simulink model "HDVSim_BEV_WPT_Truck"  (R2018b)
settings: Solver=ode3  StartTime=0.0  StopTime=inf  FixedStep=sample_time  MaxStep=0.1  RelTol=1e-3  EnableMultiTasking=on
model workspace (MAT data in the model file):
  Anschaffungskosten = [3707764736; 2; 1; 1; 1; 1]
  Bat.Useable_range = 1
  Bat.Useable_capacity = 400
  Bat.Type = 2
  Bat.SOC_start = 1
  Bat.Voltage = 800
  Bat.Efficiency_power_electronics = 0.95
  Bat.Charge_cycles = 2274.15994574
  DrvTrn = 12
  Eigenschaften = [3707764736; 2; 1; 1; 2; 2]
  Electric_Truck = 1
  Elektro_LKW = 1
  Fahrzyklus = 2
  FuelCell.P_nom = 0
  Fueltype = 12
  Gewichte = [3707764736; 2; 1; 1; 3; 3]
  Hybrid_LKW = 1
  Hybrid_Truck = 1
  IZ12 = 3600
  Kraftstoff = 12
  SOC_T_EM_completely_available = 0.5
  SOC_addition = 0.4
  SOC_target = 0.85
  Sailing_without_drag_torque = 1
  TCO = TCO
  TCO.Verhaeltnis_Anh_Lkw = [1 1]
  TCO.Kaufpreis = [141995 59191]
  TCO.Preisanstieg_Wiederbeschaffung = [3.5 3.5]
  TCO.Wiederbeschaffungsneupreis = [96696 54183]
  TCO.Restwert = [42599 0]
  TCO.CNG_Verbrauch = 0
  TCO.LNG_Verbrauch = 0
  TCO.Wasserstoffverbrauch = 0
  TCO.Reifenkosten_Satz = [2700 5008]
  TCO.Abschreibungswert = [118864 81874]
  TCO.Mautsatz = 0
  TCO.Tagesarbeitszeit = 8
  TCO.Sonstige_Arbeiten_Tag = 0.5
  TCO.Bruttostundenlohn_Fahrer = 13
  TCO.Einsatztage_Jahr = 240
  TCO.Jahresbruttolohn_Fahrer = 24960
  TCO.Personalfaktor = 1.3
  TCO.Jahresbruttolohn_FZG = 32448
  TCO.Sozialaufwendungen_Proz = 25.75
  TCO.Sozialaufwendungen_EUR = 8355
  TCO.Aushilfsfahrer = 0
  TCO.Spesen_Tag = 12
  TCO.Spesen_Jahr = 2880
  TCO.Fahrerkosten_Sonstige = 0
  TCO.Fahrpersonalkosten_Summe = 43683
  TCO.Dieselpreis = 0.95
  TCO.Dieselpreis_Fremd = 1
  TCO.Adbluepreis = 0.68
  TCO.CNGpreis = 0.88
  TCO.LNGpreis = 0.81
  TCO.Strompreis = 0.12
  TCO.Wasserstoffpreis = 9
  TCO.Verzinsung_Umlaufvermoegen = 8.4
  TCO.Verzinsung_Anlagevermoegen = 8.4
  TCO.Verwaltungskosten = 10.7
  TCO.Fahrtstrecke = 358.120895046
  TCO.Anteil_Mautstrecke = 75
  TCO.Beladezeit_Gespann = 0.25
  TCO.Entladezeit_Gespann = 0.25
  TCO.Anteil_Leerfahrten = 0
  TCO.Durchschnittsgeschwindigkeit = 75.7215565703
  TCO.Tageslenkzeit = 6.78
  TCO.Jahresfahrleistung = [123214 123214]
  TCO.Nutzungsdauer = [6 12]
  TCO.Reifenfahrleistung = [140000 170000]
  TCO.Anteil_Eigentankung_Diesel = 75
  TCO.Schmierstoffverbauch = 1
  TCO.Adblueverbrauch = 4
  TCO.Afa_zeitabhaengig = [100 100]
  TCO.Afa_leistungsabhaengig = [0 0]
  TCO.Umlaufvermoegen = [6000 1400]
  TCO.Steuern = [556 373]
  TCO.Versicherung_Kfz = [6298 677]
  TCO.Versicherung_Transport = 1000
  TCO.Kommunikation = 600
  TCO.Reparaturen_Wartung_Pflege = [0.13 0.025]
  TCO.Abschreibung_zeitabh = [19811 6823]
  TCO.Umlaufvermoegen_verzinst = 622
  TCO.Anlagevermoegen_verzinst = [5964 2486]
  TCO.Versicherung = [7298 677]
  TCO.Summe_Fzg_Kosten = [34851 10359]
  TCO.Summe_fixe_Kosten = [78534 10359]
  TCO.Abschreibung_leistungsabh = [0 0]
  TCO.Reparaturkosten = [16018 3080]
  TCO.Reifenkosten = [2376 3630]
  TCO.Lebensdauer_Batteriepack = 4.74658995879
  TCO.Anschaffungspreis_Akkupack = 65171.4590287
  TCO.Verschleisskosten_Batteriepack = 65171
  TCO.Summe_variable_Kosten = [83565 6710]
  TCO.Summe_fixe_variable_Kosten = [162099 17069]
  TCO.Gemeinkosten = 21468
  TCO.Mautkosten = 0
  TCO.Gesamtkosten = 200636
  TCO.Ergebnis = table
  TCO.Ergebnis.data = {{[]; 26634; 622; 8450; 929; 7975; 600; 45210; 43683; 88893; []; 0; 0; 0; 19098; 0; 6006; 90275; []; 179168; 21468; 0; 200636}, {[]; 0.216160501242; 0.00504812764783; 0.0685798691707; 0.00753972762835; 0.0647247877676; 0.00486957650916; 0.366922589965; 0.35452951775; 0.721452107715; []; 0; 0; 0; 0.154998620287; 0; 0.0487444608567; 0.732668365608; []; 1.45412047332; 0.174233447498; 0; 1.62835392082}, {[]; []; []; []; []; []; []; []; []; []; []; []; []; []; []; []; []; []; []; []; []; []; []}, {'#cccccc'; '#9DFF00'; '#CEFF00'; '#FFFF00'; '#FFDF00'; '#FFB900'; '#FF9200'; '#FF5D00'; '#FF7F7F'; '#FF2900'; '#cccccc'; '#00FF85'; '#00FFC6'; '#00FFF2'; '#00FFFF'; '#00DBFF'; '#00B3FF'; '#0088FF'; '#cccccc'; '#B100FF'; '#FF00FF'; '#FF00C0'; '#FF0070'}, [0; 1; 1; 1; 1; 1; 1; 0; 1; 0; 0; 1; 1; 1; 1; 1; 1; 0; 0; 0; 1; 1; 0]}
  TCO.Ergebnis.ndims = 2
  TCO.Ergebnis.nrows = 23
  TCO.Ergebnis.rownames = {'Zeitabh_Fzg_Kosten'; 'Abschreibung_zeitabh'; 'Umlaufvermoegen_verzinst'; 'Anlagevermoegen_verzinst'; 'Steuern'; 'Versicherung'; 'Kommunikation'; 'Summe_Fzg_Kosten'; 'Fahrpersonalkosten_Summe'; 'Summe_fixe_Kosten'; 'Leistungsabh_Fzg_Kosten'; 'Abschreibung_leistungsabh'; 'Kraftstoffkosten'; 'Adbluekosten'; 'Reparaturkosten'; 'Schmierstoffkosten'; 'Reifenkosten'; 'Summe_variable_Kosten'; 'Summe_Kosten'; 'Summe_fixe_variable_Kosten'; 'Gemeinkosten'; 'Mautkosten'; 'Gesamtkosten'}
  TCO.Ergebnis.nvars = 5
  TCO.Ergebnis.varnames = {'Euro_Jahr', 'Euro_km', 'Prozent', 'Cellcolor', 'Diagramm'}
  TCO.Ergebnis.props.CustomProps = struct()
  TCO.Ergebnis.props.VariableCustomProps = struct()
  TCO.Ergebnis.props.versionSavedFrom = 3
  TCO.Ergebnis.props.minCompatibleVersion = 1
  TCO.Ergebnis.props.incompatibilityMsg = ''
  TCO.Ergebnis.props.VersionSavedFrom = 3
  TCO.Ergebnis.props.Description = ''
  TCO.Ergebnis.props.DimensionNames = {'Row', 'Variables'}
  TCO.Ergebnis.props.UserData = []
  TCO.Ergebnis.props.VariableDescriptions = {}
  TCO.Ergebnis.props.VariableUnits = {}
  TCO.Ergebnis.props.VariableContinuity = {}
  TCO_Anhaenger = 1
  TCO_Trailer = 1
  T_distance_LPS_down = 250
  T_distance_LPS_up = 250
  Temp1 = [1000 1100 1200 1300 1400 1500 1600 1700 1800 1900 2000 2100 2200 2300 2400 2500 2600 2700 2800 2900 3000 3100 3200 3300 3400 3500 3600 3700 3800 3900 4000 4100 4200 4300 4400 4500 4600 4700 4800 4900 5000]
  VSim.Display = 3
  VSim.Entwurf = 0
  VSim.Opt = 0
  VSim.v_t = [0 0 0 0 0 0 7.99755670495 16.5932528737 22.7161932573 27.4198466579 27.9147182661 29.6860764389 33.4325730161 36.7505814021 39.7508189942 42.5028973365 44.7631603047 44.2850927904 46.12750995 48.4837783408 50.7030690616 52.8034153875 54.7991287178 56.7017938485 58.520948941 60.2645598986 61.9393623324 63.551113455 65.1047825506 66.6046953402 68.0546450626 69.4579815644 70.8176809608 72.1364027813 72.1303435542 72.2830929427 73.5794997033 74.8382617013 76.0614012431 77.2507716609 78.4080631952 79.5348241022 80.6324765407 81.7023309876 82.7455984026 83.7634008372 84.7567809142 85.7267097376 86.6740926498 86.812753067 86.4835070605 86.3865018146 86.3615004533]
  Vehicle = 'BEV_WPT'
  Vorausschauender_Tempomat = 1
  WPT.Voltage = 800
  WPT.SOC_target = 0.8
  WPT.SOC_electric_only = 0.3
  WPT.P_max = 200
  WPT.eta = 0.88
  WPT.expansion = 0.43
  acquisition_costs = [3707764736; 2; 1; 1; 3; 3]
  addition_for_critical_slope = 0.01
  ambient.gravity = 9.80665
  ambient.air_density = 1.1883
  ambient.friction_coeff = 0.8
  auxiliary_consumers.onBattery = 0
  auxiliary_consumers.power = 3500
  critical_altitude_difference = 50
  cycle.distance = [0 1 2 3 4 5 6 7 8 9 10 11 ... (358139 elements, 358139x1)]
  cycle.speed = [21.8055555556 21.8055555556 21.8055555556 21.8055555556 21.8055555556 21.8055555556 21.8055555556 21.8055555556 21.8055555556 21.8055555556 21.8055555556 21.8055555556 ... (358139 elements, 358139x1)]
  cycle.slope = [-0.0160892295393 -0.0161214571277 -0.0161536847161 -0.0161859123044 -0.0162181398928 -0.0162503674812 -0.0162825950696 -0.016314822658 -0.0163470502464 -0.0163792778348 -0.0164115054232 -0.0164437330115 ... (358139 elements, 358139x1)]
  cycle.stop_time = [0 0 0 0 0 0 0 0 0 0 0 0 ... (358139 elements, 358139x1)]
  cycle.altitude = [558.98391077 558.967789313 558.951635629 558.935449716 558.919231576 558.902981209 558.886698614 558.870383791 558.854036741 558.837657463 558.821245958 558.804802225 ... (358139 elements, 358139x1)]
  cycle.stop_end = 0
  cycle.stop_start = 0
  cycle.speed_init = 0
  cycle.delta12 = [0 0 0 0 0 0 0 0 0 0 0 0 ... (358139 elements, 1x358139)]
  cycle.delta23 = [0 0 0 0 0 0 0 0 0 0 0 0 ... (358139 elements, 1x358139)]
  dcycle = 2
  distance_1_PPC = 175
  distance_2_PPC = 200
  distance_PPC_altitude_difference = 10000
  driver.controller_proportional_gain = 230000
  driver.controller_integral_gain = 3000
  driver.slew_rate_throttle_in = 2
  driver.slew_rate_throttle_out = -2
  driver.slew_rate_brake_in = 2
  driver.slew_rate_brake_out = -2
  em.M_max = 1563
  em.n_max = 10000
  em.n_eck = 2200
  em.P_max = 360.089349954
  em.shift_parameter.n_pref = 2068
  em.shift_parameter.n2 = 462
  em.shift_parameter.n6 = 8000
  em.shift_parameter.M_max = 1563
  em.fuel.co2_per_kwh = 504
  em.speed = [0 10 20 30 40 50 60 70 80 90 100 110 ... (1001 elements, 1x1001)]
  em.trq = [1563 1563 1563 1563 1563 1563 1563 1563 1563 1563 1563 1563 ... (1001 elements, 1x1001)]
  em.Type = 2
  em.efficiency.characteristic_map = [0.001 0.001 0.001 0.001 0.001 0.001 0.001 0.001 0.001 0.001 0.001 0.001 ... (20301 elements, 101x201)]
  em.efficiency.speed = [0 50 100 150 200 250 300 350 400 450 500 550 600 650 700 750 800 850 900 950 1000 1050 1100 1150 1200 1250 1300 1350 1400 1450 1500 1550 1600 1650 1700 1750 1800 1850 1900 1950 2000 2050 2100 2150 2200 2250 2300 2350 2400 2450 2500 2550 2600 2650 2700 2750 2800 2850 2900 2950 3000 3050 3100 3150 3200 3250 3300 3350 3400 3450 3500 3550 3600 3650 3700 3750 3800 3850 3900 3950 4000 4050 4100 4150 4200 4250 4300 4350 4400 4450 4500 4550 4600 4650 4700 4750 4800 4850 4900 4950 5000 5050 5100 5150 5200 5250 5300 5350 5400 5450 5500 5550 5600 5650 5700 5750 5800 5850 5900 5950 6000 6050 6100 6150 6200 6250 6300 6350 6400 6450 6500 6550 6600 6650 6700 6750 6800 6850 6900 6950 7000 7050 7100 7150 7200 7250 7300 7350 7400 7450 7500 7550 7600 7650 7700 7750 7800 7850 7900 7950 8000 8050 8100 8150 8200 8250 8300 8350 8400 8450 8500 8550 8600 8650 8700 8750 8800 8850 8900 8950 9000 9050 9100 9150 9200 9250 9300 9350 9400 9450 9500 9550 9600 9650 9700 9750 9800 9850 9900 9950 10000]
  em.efficiency.torque = [0 15.63 31.26 46.89 62.52 78.15 93.78 109.41 125.04 140.67 156.3 171.93 187.56 203.19 218.82 234.45 250.08 265.71 281.34 296.97 312.6 328.23 343.86 359.49 375.12 390.75 406.38 422.01 437.64 453.27 468.9 484.53 500.16 515.79 531.42 547.05 562.68 578.31 593.94 609.57 625.2 640.83 656.46 672.09 687.72 703.35 718.98 734.61 750.24 765.87 781.5 797.13 812.76 828.39 844.02 859.65 875.28 890.91 906.54 922.17 937.8 953.43 969.06 984.69 1000.32 1015.95 1031.58 1047.21 1062.84 1078.47 1094.1 1109.73 1125.36 1140.99 1156.62 1172.25 1187.88 1203.51 1219.14 1234.77 1250.4 1266.03 1281.66 1297.29 1312.92 1328.55 1344.18 1359.81 1375.44 1391.07 1406.7 1422.33 1437.96 1453.59 1469.22 1484.85 1500.48 1516.11 1531.74 1547.37 1563]
  em_M_max = 1563
  engine.shift_parameter.n_lo = 978
  engine.shift_parameter.n_pref = 1339
  engine.M_max = 0
  engine.number = 4
  final_drive.ratio = 10.0500050682
  final_drive.trq_eff = 0.98
  ifElectric = 1
  ifOptimized = 1
  look_ahead_PPC = 250
  max_distance = 358138
  name = 'Verbrauchssimulation_Elektro_WPT_LKW'
  propertie = [3707764736; 2; 1; 1; 4; 4]
  prospective_cruise_control = 1
  sample_time = 0.1
  tank.v_diesel = 0
  tank.v_lng = 0
  tank.v_cng = 0
  tank.m_h2 = 0
  tires.radius = 0.4465
  tires.roll_drag_coeff.total = 0.0043
  transmission.init_gear = 1
  transmission.shift_time = 1
  transmission.ratios = [2.66167387947 1.631463723 1 0.612946512939]
  transmission.trq_eff = [0.96 0.97 0.98 0.99]
  transmission.lamda = [1.0669857778 1.02376767201 1.01308044742 1.00911164176]
  transmission.z = 4
  v_PPC_delta = 2.77777777778
  v_max_electrical_drive_only = 13.8888888889
  vehicle.long_truck = 0
  vehicle.payload = 19300
  vehicle.frontal_area = 10.2
  vehicle.air_drag_coeff = 0.53
  vehicle.Fx_max = 90221.18
  vehicle.F_brake_max = 309890.14
  vehicle.mass = 13863.4845097
  vehicle_F_brake_max = 309890.14
  weights = weights
  weights.m_Gearbox = 146.265985714
  weights.m_Retarder = 82
  weights.m_Battery = 1987.57763975
  weights.m_EM = 400.099277727
  weights.m_PwrElectr = 33.3416064773
  weights.m_Charger = 591
  weights.m_Trailer = 5400
  weights.m_Total = 8463.48450967
  weights.m_Base = 5223.2
  weights.Electric = 1
  weights.Hybrid = 1
  weights.max_Payload = 27136.5154903
  weights.m_Max = 41000
  x = [1563 13 1 400 10.0500050682 4.34242437682 3 1 0 978 1339 2 2 0.8 0.3]
values from neighbouring files (symbols this model uses):
  transmission = Transmission_gearing(15.86, 7, 0, 0, final_drive.ratio)    [Parameterizing.m]
undefined symbols (not defined in the file or in its folder): Ion, Lithium
libraries: simulink x22, powerlib x4, electricdrivelib x1
(part 1 of 3)
settings Model: Creator=Alexander Suessmann  LastModifiedBy=ga73rep
settings DataIOCC: SaveFormat=Array  SignalLoggingSaveFormat=ModelDataLogs  SaveOutput=off  SignalLogging=off  DSMLogging=off  SignalLoggingName=datalog
settings OptimizationCC: ExpressionDepthLimit=2147483647  InitFltsAndDblsToZero=on  LifeSpan=inf
settings DebuggingCC: UnderspecifiedInitializationDetection=Classic  MergeDetectMultiDrivingBlocksExec=none  SolverPrmCheckMsg=warning  MultiTaskDSMMsg=warning  MultiTaskCondExecSysMsg=none  FcnCallInpInsideContextMsg=warning  AllowSymbolicDim=off  StateNameClashWarn=warning  LibraryContextMissingDiagnostic=none  SFUnexpectedBacktrackingDiag=warning  SFNoUnconditionalDefaultTransitionDiag=warning  SFExecutionAtInitializationDiag=none
settings HardwareCC: ProdBitPerPointer=32  ProdBitPerSizeT=32  ProdBitPerPtrDiffT=32  ProdLargestAtomicFloat=None  ProdIntDivRoundTo=Undefined  ProdEndianess=Unspecified  ProdWordSize=32  ProdHWDeviceType=32-bit Generic
settings ModelReferenceCC: PropagateSignalLabelsOutOfModel=off
settings SFSimCC: SimUseLocalCustomCode=off
settings RTWCC: RTWUseLocalCustomCode=off
settings CodeAppCC: StateflowObjectComments=on
settings GRTTargetCC: TargetLangStandard=C89/C90 (ANSI)  GeneratePreprocessorConditionals=Disable all  CombineOutputUpdateFcns=off  GRTInterface=on
settings ConfigComp: CovMetricSettings=dw  CovHtmlReporting=on  CovSaveCumulativeToWorkspaceVar=on  CovSaveSingleToWorkspaceVar=on  CovExternalEMLEnable=off  CovSFcnEnable=off
settings SimscapeCC: SimscapeNormalizeSystem=off

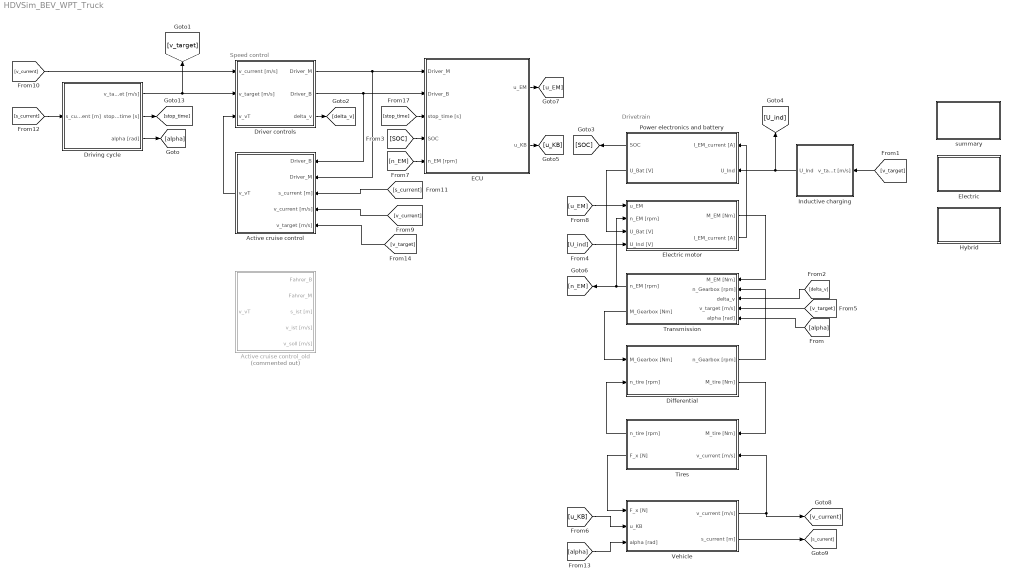
== system HDVSim_BEV_WPT_Truck ==
  note: Drivetrain
  note: Speed control
  Active cruise control : Subsystem
  Active cruise control_old : Subsystem  Commented=on
  Differential : Subsystem
  Driver controls : Subsystem
  Driving cycle : Subsystem
  ECU : Subsystem
  Electric : Subsystem
  Electric motor : Subsystem
  Hybrid : Subsystem
  Inductive charging : Subsystem
  Power electronics and battery : Subsystem
  Tires : Subsystem
  Transmission : Subsystem
  Vehicle : Subsystem
  summary : Subsystem
  wires:
  Vehicle/1(v_current [m/s]) -> [v_current], Tires/2(v_current [m/s])
  Active cruise control/1(v_vT) -> Driver controls/3(v_vT)
  Driver controls/1(Driver_M) -> Active cruise control/2(Driver_M), ECU/1(Driver_M)
  Driver controls/2(Driver_B) -> Active cruise control/1(Driver_B), ECU/2(Driver_B)
  [v_target] -> Inductive charging/1(v_target [m/s])
  Inductive charging/1(U_Ind) -> Power electronics and battery/2(U_Ind), [U_ind]
  Transmission/2(M_Gearbox [Nm]) -> Differential/1(M_Gearbox [Nm])
  Differential/1(n_Gearbox [rpm]) -> Transmission/2(n_Gearbox [rpm])
  Electric motor/1(M_EM [Nm]) -> Transmission/1(M_EM [Nm])
  Electric motor/2(I_EM_current [A]) -> Power electronics and battery/1(I_EM_current [A])
  [alpha] -> Transmission/5(alpha [rad])
  [delta_v] -> Transmission/3(delta_v)
  Power electronics and battery/1(SOC) -> [SOC]
  [SOC] -> ECU/4(SOC)
  Power electronics and battery/2(U_Bat [V]) -> Electric motor/3(U_Bat [V])
  Transmission/1(n_EM [rpm]) -> [n_EM], Electric motor/2(n_EM [rpm])
  [U_ind] -> Electric motor/4(U_Ind [V])
  [v_target] -> Transmission/4(v_target [m/s])
  Differential/2(M_tire [Nm]) -> Tires/1(M_tire [Nm])
  Tires/1(n_tire [rpm]) -> Differential/2(n_tire [rpm])
  Tires/2(F_x [N]) -> Vehicle/1(F_x [N])
  ECU/2(u_KB) -> [u_KB]
  [u_KB] -> Vehicle/2(u_KB)
  [n_EM] -> ECU/5(n_EM [rpm])
  ECU/1(u_EM) -> [u_EM]
  [u_EM] -> Electric motor/1(u_EM)
  [v_current] -> Active cruise control/4(v_current [m/s])
  [v_current] -> Driver controls/1(v_current [m/s])
  Vehicle/2(s_current [m]) -> [s_current]
  [s_current] -> Active cruise control/3(s_current [m])
  [s_current] -> Driving cycle/1(s_current [m])
  Driving cycle/3(alpha [rad]) -> [alpha]
  [alpha] -> Vehicle/3(alpha [rad])
  Driving cycle/1(v_target [m/s]) -> [v_target], Driver controls/2(v_target [m/s])
  [v_target] -> Active cruise control/5(v_target [m/s])
  Driving cycle/2(stop_time [s]) -> [stop_time]
  [stop_time] -> ECU/3(stop_time [s])
  Driver controls/3(delta_v) -> [delta_v]

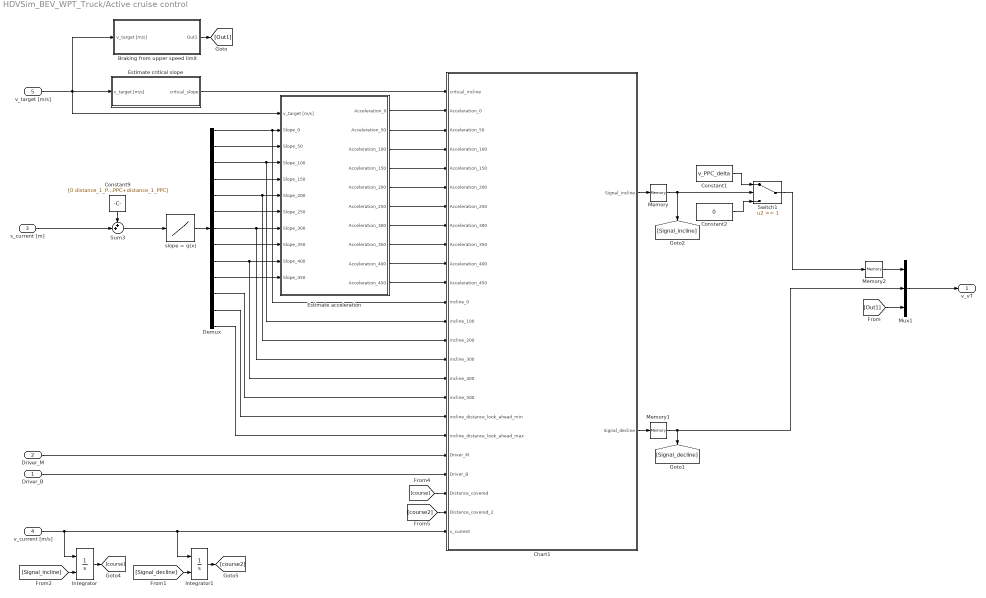
== system HDVSim_BEV_WPT_Truck/Active cruise control ==
  Driver_B : Inport
  Driver_M : Inport  Port=2
  s_current [m] : Inport  Port=3
  v_current [m/s] : Inport  Port=4
  v_target [m/s] : Inport  Port=5
  Braking from upper speed limit : Subsystem
  Chart1 : Stateflow chart  TreatAsAtomicUnit=on
  Constant1 : Constant  Value=v_PPC_delta
  Constant2 : Constant  Value=0
  Constant9 : Constant  Value=[0 distance_1_PPC/5 2*distance_1_PPC/5 3*distance_1_PPC/5 4*distance_1_PPC/5 distance_1_PPC 6*distance_1_PPC/5 7*distance_1_PPC/5 8*distance_1_PPC/5 9*distance_1_PPC/5 10*distance_1_PPC/5 look_ahead_PPC look_ahead_PPC+distance_1_PPC]
  Demux : Demux  Outputs=13
  Estimate acceleration : Subsystem
  Estimate critical slope : Subsystem
  Integrator : Integrator  ExternalReset=rising
  Integrator1 : Integrator  ExternalReset=rising
  Memory : Memory
  Memory1 : Memory
  Memory2 : Memory
  Mux1 : Mux  Inputs=3
  Sum3 : Sum  Inputs=++|
  Switch1 : Switch  Threshold=1
  slope = q(x) : Lookup  InputValues=cycle.distance  Table=cycle.slope  LookUpMeth=Interpolation-Use End Values
  v_vT : Outport
  wires:
  slope = q(x)/1 -> Demux/1
  Demux/1 -> Chart1/12(incline_0), Estimate acceleration/2(Slope_0)
  Estimate critical slope/1(critical_slope) -> Chart1/1(critical_incline)
  Chart1/1(Signal_incline) -> Memory/1
  v_current [m/s]/1 -> Integrator/1, Chart1/24(v_current), Integrator1/1
  Memory/1 -> [Signal_incline], Switch1/2
  Mux1/1 -> v_vT/1
  Constant9/1 -> Sum3/1
  Sum3/1 -> slope = q(x)/1
  Estimate acceleration/1(Acceleration_0) -> Chart1/2(Acceleration_0)
  Estimate acceleration/2(Acceleration_50) -> Chart1/3(Acceleration_50)
  Estimate acceleration/3(Acceleration_100) -> Chart1/4(Acceleration_100)
  Estimate acceleration/4(Acceleration_150) -> Chart1/5(Acceleration_150)
  Estimate acceleration/5(Acceleration_200) -> Chart1/6(Acceleration_200)
  Estimate acceleration/6(Acceleration_250) -> Chart1/7(Acceleration_250)
  Estimate acceleration/7(Acceleration_300) -> Chart1/8(Acceleration_300)
  Estimate acceleration/8(Acceleration_350) -> Chart1/9(Acceleration_350)
  Chart1/2(Signal_decline) -> Memory1/1
  Memory1/1 -> Mux1/2, [Signal_decline]
  Driver_B/1 -> Chart1/21(Driver_B)
  Estimate acceleration/9(Acceleration_400) -> Chart1/10(Acceleration_400)
  Estimate acceleration/10(Acceleration_450) -> Chart1/11(Acceleration_450)
  Driver_M/1 -> Chart1/20(Driver_M)
  Demux/2 -> Estimate acceleration/3(Slope_50)
  Demux/3 -> Chart1/13(incline_100), Estimate acceleration/4(Slope_100)
  Demux/4 -> Estimate acceleration/5(Slope_150)
  Demux/5 -> Chart1/14(incline_200), Estimate acceleration/6(Slope_200)
  Demux/6 -> Estimate acceleration/7("Slope_250 ")
  Demux/7 -> Chart1/15(incline_300), Estimate acceleration/8(Slope_300)
  Demux/8 -> Estimate acceleration/9(Slope_350)
  Demux/9 -> Chart1/16(incline_400), Estimate acceleration/10(Slope_400)
  Demux/10 -> Estimate acceleration/11(Slope_450)
  Demux/12 -> Chart1/18(incline_distance_look_ahead_min)
  Demux/13 -> Chart1/19(incline_distance_look_ahead_max)
  v_target [m/s]/1 -> Braking from upper speed limit/1(v_target [m/s]), Estimate acceleration/1(v_target [m/s]), Estimate critical slope/1(v_target [m/s])
  Memory2/1 -> Mux1/1
  Constant1/1 -> Switch1/1
  Constant2/1 -> Switch1/3
  Demux/11 -> Chart1/17(incline_500)
  Switch1/1 -> Memory2/1
  s_current [m]/1 -> Sum3/2
  [Signal_decline] -> Integrator1/2
  Integrator1/1 -> [course2]
  Integrator/1 -> [course]
  [Signal_incline] -> Integrator/2
  [course] -> Chart1/22(Distance_covered)
  [course2] -> Chart1/23(Distance_covered_2)
  Braking from upper speed limit/1(Out1) -> [Out1]
  [Out1] -> Mux1/3

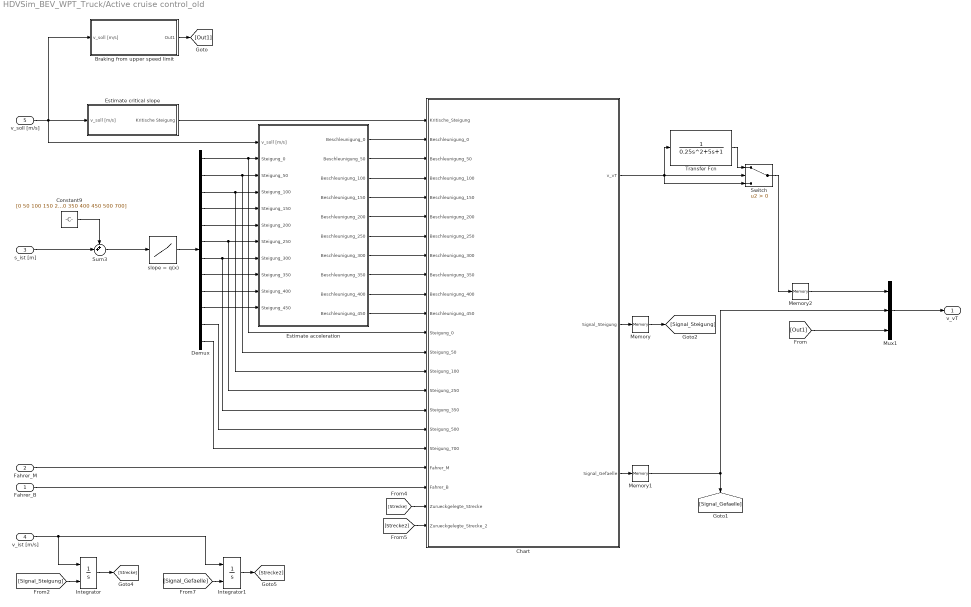
== system HDVSim_BEV_WPT_Truck/Active cruise control_old ==
  Fahrer_B : Inport
  Fahrer_M : Inport  Port=2
  s_ist [m] : Inport  Port=3
  v_ist [m/s] : Inport  Port=4
  v_soll [m/s] : Inport  Port=5
  Braking from upper speed limit : Subsystem
  Chart : Stateflow chart  TreatAsAtomicUnit=on
  Constant9 : Constant  Value=[0 50 100 150 200 250 300 350 400 450 500 700]
  Demux : Demux  Outputs=12
  Estimate acceleration : Subsystem
  Estimate critical slope : Subsystem
  Integrator : Integrator  ExternalReset=rising
  Integrator1 : Integrator  ExternalReset=rising
  Memory : Memory
  Memory1 : Memory
  Memory2 : Memory
  Mux1 : Mux  Inputs=3
  Sum3 : Sum  Inputs=++|
  Switch : Switch  Criteria=u2 > Threshold
  Transfer Fcn : TransferFcn  Denominator=[0.25 5 1]
  slope = q(x) : Lookup  InputValues=cycle.distance  Table=cycle.slope  LookUpMeth=Interpolation-Use End Values
  v_vT : Outport
  wires:
  slope = q(x)/1 -> Demux/1
  Demux/1 -> Estimate acceleration/2(Steigung_0), Chart/12(Steigung_0)
  Estimate critical slope/1(Kritische Steigung) -> Chart/1(Kritische_Steigung)
  Chart/2(Signal_Steigung) -> Memory/1
  Mux1/1 -> v_vT/1
  Constant9/1 -> Sum3/1
  s_ist [m]/1 -> Sum3/2
  Sum3/1 -> slope = q(x)/1
  Estimate acceleration/1(Beschleunigung_0) -> Chart/2(Beschleunigung_0)
  Estimate acceleration/2(Beschleunigung_50) -> Chart/3(Beschleunigung_50)
  Estimate acceleration/3(Beschleunigung_100) -> Chart/4(Beschleunigung_100)
  Estimate acceleration/4(Beschleunigung_150) -> Chart/5(Beschleunigung_150)
  Estimate acceleration/5(Beschleunigung_200) -> Chart/6(Beschleunigung_200)
  Estimate acceleration/6(Beschleunigung_250) -> Chart/7(Beschleunigung_250)
  Estimate acceleration/7(Beschleunigung_300) -> Chart/8(Beschleunigung_300)
  Estimate acceleration/8(Beschleunigung_350) -> Chart/9(Beschleunigung_350)
  Chart/3(Signal_Gefaelle) -> Memory1/1
  Memory1/1 -> [Signal_Gefaelle], Mux1/2
  Fahrer_B/1 -> Chart/20(Fahrer_B)
  Estimate acceleration/9(Beschleunigung_400) -> Chart/10(Beschleunigung_400)
  Estimate acceleration/10(Beschleunigung_450) -> Chart/11(Beschleunigung_450)
  Fahrer_M/1 -> Chart/19(Fahrer_M)
  Demux/2 -> Estimate acceleration/3(Steigung_50), Chart/13(Steigung_50)
  Demux/3 -> Estimate acceleration/4(Steigung_100), Chart/14(Steigung_100)
  Demux/4 -> Estimate acceleration/5(Steigung_150)
  Demux/5 -> Estimate acceleration/6(Steigung_200)
  Demux/6 -> Estimate acceleration/7("Steigung_250 "), Chart/15(Steigung_250)
  Demux/7 -> Estimate acceleration/8(Steigung_300), Chart/16(Steigung_350)
  Demux/8 -> Estimate acceleration/9(Steigung_350)
  Demux/9 -> Estimate acceleration/10(Steigung_400)
  Demux/10 -> Estimate acceleration/11(Steigung_450)
  Demux/11 -> Chart/17(Steigung_500)
  Demux/12 -> Chart/18(Steigung_700)
  v_soll [m/s]/1 -> Braking from upper speed limit/1(v_soll [m/s]), Estimate acceleration/1(v_soll [m/s]), Estimate critical slope/1(v_soll [m/s])
  Memory2/1 -> Mux1/1
  Switch/1 -> Memory2/1
  Chart/1(v_vT) -> Switch/3, Switch/2, Transfer Fcn/1
  Transfer Fcn/1 -> Switch/1
  Braking from upper speed limit/1(Out1) -> [Out1]
  [Out1] -> Mux1/3
  Memory/1 -> [Signal_Steigung]
  v_ist [m/s]/1 -> Integrator1/1, Integrator/1
  Integrator/1 -> [Strecke]
  [Signal_Gefaelle] -> Integrator1/2
  [Signal_Steigung] -> Integrator/2
  Integrator1/1 -> [Strecke2]
  [Strecke] -> Chart/21(Zurueckgelegte_Strecke)
  [Strecke2] -> Chart/22(Zurueckgelegte_Strecke_2)

== system HDVSim_BEV_WPT_Truck/Differential ==
  note: Effective wheel torque
  M_Gearbox [Nm] : Inport
  n_tire [rpm] : Inport  Port=2
  Differential efficiency : Constant  Value=final_drive.trq_eff
  Differential gear ratio : Constant  Value=final_drive.ratio
  M' = M*i*eta : Product  Inputs=***
  Product : Product  Inputs=/  RndMeth=Zero
  Switch : Switch  ZeroCross=off
  n' *i = n : Product  RndMeth=Zero
  n_Gearbox [rpm] : Outport
  M_tire [Nm] : Outport  Port=2
  wires:
  M_Gearbox [Nm]/1 -> M' = M*i*eta/1, Switch/2
  n_tire [rpm]/1 -> n' *i = n/2
  Differential gear ratio/1 -> n' *i = n/1, M' = M*i*eta/3
  n' *i = n/1 -> n_Gearbox [rpm]/1
  M' = M*i*eta/1 -> M_tire [Nm]/1
  Differential efficiency/1 -> Product/1, Switch/1
  Product/1 -> Switch/3
  Switch/1 -> M' = M*i*eta/2

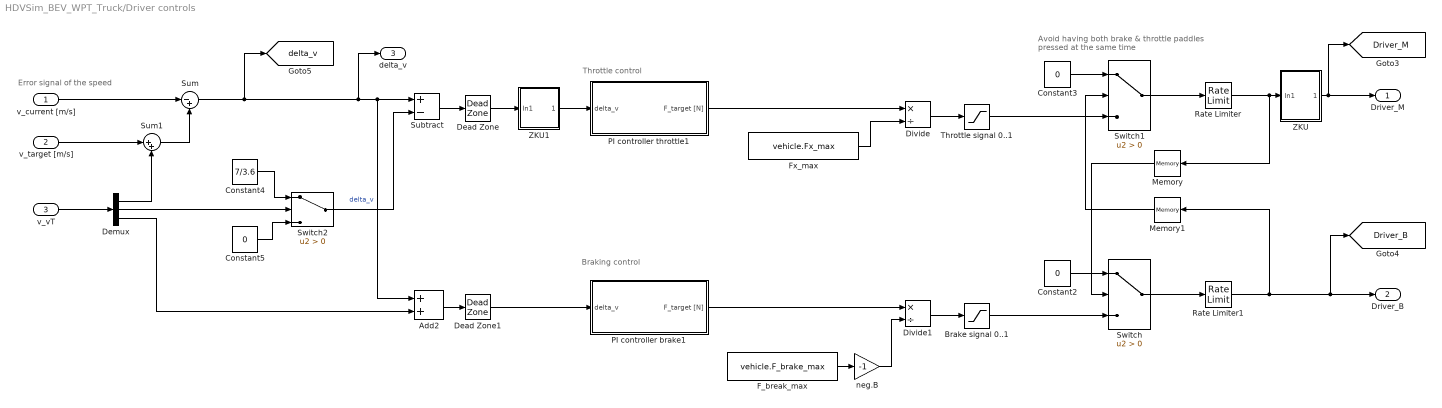
== system HDVSim_BEV_WPT_Truck/Driver controls ==
  note: Error signal of the speed
  note: Throttle control
  note: Braking control
  note: Avoid having both brake & throttle
      | paddles pressed at the same time
  v_current [m/s] : Inport
  v_target [m/s] : Inport  Port=2
  v_vT : Inport  Port=3
  Add2 : Sum
  Brake signal 0..1 : Saturate  UpperLimit=1  LowerLimit=0  ZeroCross=off
  Constant2 : Constant  Value=0
  Constant3 : Constant  Value=0
  Constant4 : Constant  Value=7/3.6
  Constant5 : Constant  Value=0
  Dead Zone : DeadZone  LowerValue=-0.1/3.6  UpperValue=0.1/3.6  ZeroCross=off  SaturateOnIntegerOverflow=on
  Dead Zone1 : DeadZone  LowerValue=-0.1/3.6  UpperValue=0  ZeroCross=off  SaturateOnIntegerOverflow=on
  Demux : Demux  Outputs=3
  Divide : Product  Inputs=*/
  Divide1 : Product  Inputs=*/
  F_break_max : Constant  Value=vehicle.F_brake_max
  Fx_max : Constant  Value=vehicle.Fx_max
  Memory : Memory
  Memory1 : Memory
  PI controller brake1 : Subsystem
  PI controller throttle1 : Subsystem
  Rate Limiter : RateLimiter  RisingSlewLimit=driver.slew_rate_throttle_in  FallingSlewLimit=driver.slew_rate_throttle_out  SampleTimeMode=inherited
  Rate Limiter1 : RateLimiter  RisingSlewLimit=driver.slew_rate_brake_in*100  FallingSlewLimit=driver.slew_rate_brake_out*100  SampleTimeMode=inherited
  Subtract : Sum  Inputs=+-
  Sum : Sum  Inputs=|-+
  Sum1 : Sum  Inputs=|++
  Switch : Switch  Criteria=u2 > Threshold
  Switch1 : Switch  Criteria=u2 > Threshold
  Switch2 : Switch  Criteria=u2 > Threshold
  Throttle signal 0..1 : Saturate  UpperLimit=1  LowerLimit=0  ZeroCross=off
  ZKU : Subsystem
  ZKU1 : Subsystem
  neg.B : Gain  Gain=-1
  Driver_M : Outport
  Driver_B : Outport  Port=2
  delta_v : Outport  Port=3
  tag scope: Driver_B=global, Driver_M=global, delta_v=global
  wires:
  Fx_max/1 -> Divide/2
  neg.B/1 -> Divide1/2
  Divide1/1 -> Brake signal 0..1/1
  Divide/1 -> Throttle signal 0..1/1
  F_break_max/1 -> neg.B/1
  Switch/1 -> Rate Limiter1/1
  Brake signal 0..1/1 -> Switch/3
  Constant2/1 -> Switch/1
  Switch1/1 -> Rate Limiter/1
  Throttle signal 0..1/1 -> Switch1/3
  Rate Limiter1/1 -> Memory1/1, [Driver_B], Driver_B/1
  Rate Limiter/1 -> ZKU/1(In1), Memory/1
  Constant3/1 -> Switch1/1
  PI controller throttle1/1(F_target [N]) -> Divide/1
  PI controller brake1/1(F_target [N]) -> Divide1/1
  ZKU/1(Out1) -> [Driver_M], Driver_M/1
  Memory/1 -> Switch/2
  Memory1/1 -> Switch1/2
  Sum1/1 -> Sum/2
  v_current [m/s]/1 -> Sum/1
  Sum/1 -> [delta_v], delta_v/1, Add2/1, Subtract/1  "delta_v"
  Dead Zone/1 -> ZKU1/1(In1)
  Dead Zone1/1 -> PI controller brake1/1(delta_v)
  ZKU1/1(Out1) -> PI controller throttle1/1(delta_v)
  v_vT/1 -> Demux/1
  Switch2/1 -> Subtract/2
  Demux/2 -> Switch2/2
  Constant4/1 -> Switch2/1
  Constant5/1 -> Switch2/3
  Subtract/1 -> Dead Zone/1
  Demux/1 -> Sum1/2
  v_target [m/s]/1 -> Sum1/1
  Demux/3 -> Add2/2
  Add2/1 -> Dead Zone1/1

== system HDVSim_BEV_WPT_Truck/Driving cycle ==
  note: Lookup tables
  s_current [m] : Inport
  Clock : Clock
  Compare To Constant : simulink/Logic and Bit Operations/Compare To Constant  relop=>=  const=max_distance  OutDataTypeStr=boolean
  Constant : Constant  Value=0
  Holding time at the beginning : Subsystem
  Holding time at the end : Subsystem
  Significant speed deviation : Subsystem
  Slope-> angle : Trigonometry  Operator=atan
  Stop Simulation : Stop
  Stop Simulation 2 : Stop
  Switch : Switch  Criteria=u2 > Threshold
  Terminator : Terminator
  altitude : Lookup_n-D  NumberOfTableDimensions=1  BreakpointsForDimension1=cycle.distance  Table=cycle.altitude  InterpMethod=Flat  RndMeth=Simplest  InputSameDT=on
  slope : Lookup_n-D  NumberOfTableDimensions=1  BreakpointsForDimension1=cycle.distance  Table=cycle.slope  InterpMethod=Flat  RndMeth=Simplest  InputSameDT=on
  speed : Lookup_n-D  NumberOfTableDimensions=1  BreakpointsForDimension1=cycle.distance  Table=cycle.speed  InterpMethod=Flat  RndMeth=Simplest  InputSameDT=on
  stop_time : Lookup_n-D  NumberOfTableDimensions=1  BreakpointsForDimension1=cycle.distance  Table=cycle.stop_time  InterpMethod=Flat  RndMeth=Simplest  InputSameDT=on
  v_target [m/s] : Outport
  stop_time [s] : Outport  Port=2
  alpha [rad] : Outport  Port=3
  tag scope: altitude=global, slope_q=global, v_target_dc=global
  wires:
  slope/1 -> Slope-> angle/1, [slope_q]
  Slope-> angle/1 -> alpha [rad]/1
  Compare To Constant/1 -> Holding time at the end/1(In1)
  Clock/1 -> Terminator/1  "duration"
  Switch/1 -> [v_target_dc], v_target [m/s]/1
  s_current [m]/1 -> slope/1, Significant speed deviation/1(In1), Compare To Constant/1, altitude/1, speed/1, stop_time/1
  stop_time/1 -> stop_time [s]/1, Switch/2
  speed/1 -> Holding time at the beginning/1(In1)
  Holding time at the end/1(Out1) -> Stop Simulation/1
  Holding time at the beginning/1(Out1) -> Switch/3
  Constant/1 -> Switch/1
  altitude/1 -> [altitude]
  Significant speed deviation/1(Out1) -> Stop Simulation 2/1

== system HDVSim_BEV_WPT_Truck/ECU ==
  Driver_M : Inport
  Driver_B : Inport  Port=2
  stop_time [s] : Inport  Port=3
  SOC : Inport  Port=4
  n_EM [rpm] : Inport  Port=5
  Approach to stop : Subsystem
  Calculations for braking : Subsystem
  Calculations for throttle : Subsystem
  Chart : Stateflow chart  TreatAsAtomicUnit=on
  Saturation : Saturate  UpperLimit=1  LowerLimit=-1
  Saturation2 : Saturate  UpperLimit=1  LowerLimit=0
  Scope : Scope  NumInputPorts=6
  Sum : Sum  Inputs=++|
  Sum1 : Sum  Inputs=++|
  u_EM : Outport
  u_KB : Outport  Port=2
  tag scope: REKUP=global
  wires:
  Chart/1(u_EM) -> Saturation/1
  Chart/2(u_KB) -> Saturation2/1
  Sum1/1 -> Chart/2(Driver_B), Calculations for braking/2(Driver_B)
  Sum/1 -> Chart/1(Driver_M), Calculations for throttle/1(Driver_M)
  Calculations for braking/2(F_brake_elec_max [N]) -> Chart/7(F_brake_el_max)
  Calculations for braking/1(F_brake_required [N]) -> Chart/6(F_brake_required)
  SOC/1 -> Chart/3(SOC), Calculations for throttle/2(SOC)
  Calculations for throttle/2(M_Required [Nm]) -> Chart/5(M_required)
  Saturation2/1 -> u_KB/1, Scope/2  "u_KB"
  Chart/3(ROLL) -> Scope/3  "ROLL"
  Chart/4(REKUP) -> [REKUP], Scope/4  "REKUP"
  [slope_q] -> Scope/5  "Slope"
  Calculations for throttle/1(M_EM_max [Nm]) -> Chart/4(M_EM_max), Calculations for braking/1(M_EM_max [Nm])
  [SOC] -> Scope/6  "SOC"
  Saturation/1 -> u_EM/1, Scope/1  "u_EM"
  stop_time [s]/1 -> Approach to stop/1(stop_time [s])
  Approach to stop/2(Driver_B) -> Sum1/1
  Approach to stop/1(Driver_M) -> Sum/1
  Driver_M/1 -> Sum/2
  Driver_B/1 -> Sum1/2
  n_EM [rpm]/1 -> Calculations for throttle/3(n_EM [rpm])
  [gear] -> Calculations for braking/3(Gear)

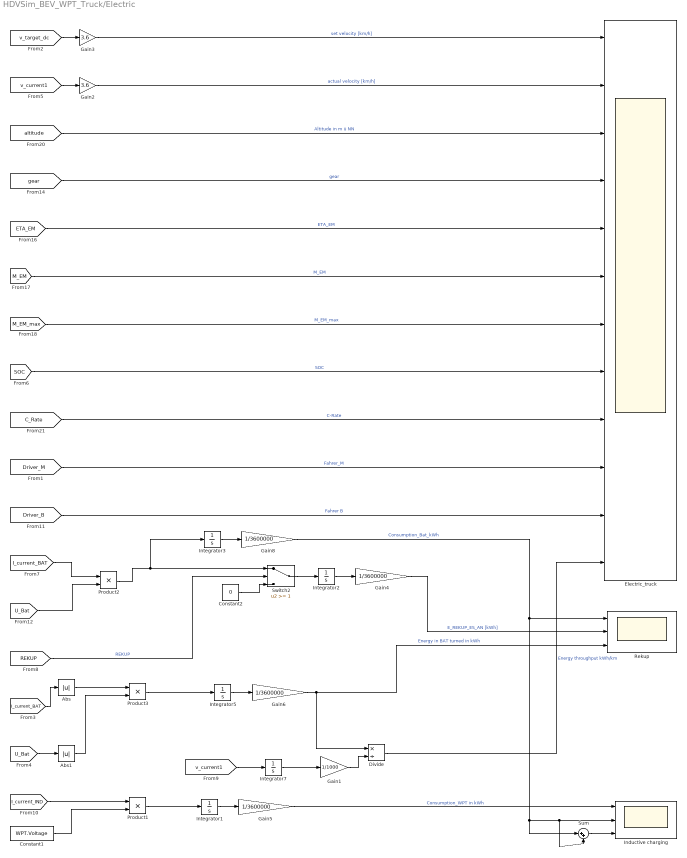
== system HDVSim_BEV_WPT_Truck/Electric ==
  Abs : Abs
  Abs1 : Abs
  Constant1 : Constant  Value=WPT.Voltage
  Constant2 : Constant  Value=0
  Divide : Product  Inputs=*/
  Electric_truck : Scope  NumInputPorts=12
  Gain1 : Gain  Gain=1/1000
  Gain2 : Gain  Gain=3.6
  Gain3 : Gain  Gain=3.6
  Gain{4..6,8} (4 alike) : Gain  Gain=1/3600000
  Inductive charging : Scope  NumInputPorts=3
  Integrator{1..3,5} (4 alike) : Integrator
  Integrator7 : Integrator  InitialCondition=0.00000001
  Product1 : Product
  Product2 : Product
  Product3 : Product
  Rekup : Scope  NumInputPorts=3
  Sum : Sum  Inputs=|++
  Switch2 : Switch  Threshold=1
  wires:
  [v_target_dc] -> Gain3/1
  Gain3/1 -> Electric_truck/1  "set velocity [km/h]"
  [v_current1] -> Gain2/1
  Gain2/1 -> Electric_truck/2  "actual velocity [km/h]"
  Gain4/1 -> Rekup/2  "E_REKUP_ES_AN [kWh]"
  Constant2/1 -> Switch2/3
  Integrator2/1 -> Gain4/1
  Switch2/1 -> Integrator2/1
  [REKUP] -> Switch2/2  "REKUP"
  [U_Bat] -> Product2/2
  [I_current_BAT] -> Product2/1
  [gear] -> Electric_truck/4  "gear"
  [ETA_EM] -> Electric_truck/5  "ETA_EM"
  [M_EM] -> Electric_truck/6  "M_EM"
  [M_EM_max] -> Electric_truck/7  "M_EM_max"
  [SOC] -> Electric_truck/8  "SOC"
  Product2/1 -> Integrator3/1, Switch2/1
  Gain8/1 -> Sum/1, Sum/2, Inductive charging/2, Rekup/1  "Consumption_Bat_kWh"
  Integrator3/1 -> Gain8/1
  [U_Bat] -> Abs1/1
  [I_current_BAT] -> Abs/1
  Product3/1 -> Integrator5/1
  Integrator5/1 -> Gain6/1
  Abs/1 -> Product3/1
  Abs1/1 -> Product3/2
  [altitude] -> Electric_truck/3  "Altitude in m ü NN"
  [C_Rate] -> Electric_truck/9  "C-Rate"
  [Driver_M] -> Electric_truck/10  "Fahrer_M"
  [Driver_B] -> Electric_truck/11  "Fahrer B"
  Gain6/1 -> Divide/1, Rekup/3  "Energy in BAT turned in kWh"
  Divide/1 -> Electric_truck/12  "Energy throughput kWh/km"
  [v_current1] -> Integrator7/1
  Integrator7/1 -> Gain1/1
  Gain1/1 -> Divide/2
  [I_current_IND] -> Product1/1
  Constant1/1 -> Product1/2
  Product1/1 -> Integrator1/1
  Gain5/1 -> Inductive charging/1  "Consumption_WPT in kWh"
  Integrator1/1 -> Gain5/1
  Sum/1 -> Inductive charging/3
